simulink model "ofdm_txrx_supermimo_coded"  (Simulink 7.1)
settings: Solver=VariableStepDiscrete  SolverMode=SingleTasking  StartTime=0.0  StopTime=2*simOnly_simLength  RelTol=1e-3
PostLoadFcn:
  | ofdm_rx_supermimo_init
InitFcn:
  | ofdm_rx_supermimo_init
StartFcn:
  | ofdm_rx_supermimo_init
values from neighbouring files (symbols this model uses):
  Action0_Config = 0* 31*2^18 + 0*2^24 + PHY_AUTORESPONSE_REQ_GOODHDR + PHY_AUTORESPONSE_REQ_GOODPKT + PHY_AUTORESPONSE_REQ_FLAGA    [ofdm_tx_supermimo_init.m]
  Action1_Config = 0* 62*2^18 + PHY_AUTORESPONSE_REQ_GOODHDR + PHY_AUTORESPONSE_REQ_GOODPKT    [ofdm_tx_supermimo_init.m]
  Action2_Config = 0    [ofdm_tx_supermimo_init.m]
  Action3_Config = 0    [ofdm_tx_supermimo_init.m]
  Action4_Config = 0    [ofdm_tx_supermimo_init.m]
  Action5_Config = 0    [ofdm_tx_supermimo_init.m]
  ActionID_AFTransmit = 31    [ofdm_tx_supermimo_init.m]
  ActionID_Disabled = 0    [ofdm_tx_supermimo_init.m]
  ActionID_SetFlagA = 62    [ofdm_tx_supermimo_init.m]
  ActionID_SetFlagB = 61    [ofdm_tx_supermimo_init.m]
  BER_RAM_init_size = 2048    [ofdm_tx_supermimo_init.m]
  channel_h = [1 0; 1 0] * 0.3    [ofdm_tx_supermimo_init.m]
  CPLength = 16    [ofdm_tx_supermimo_init.m]
  FEC_Config = fec_coding_en*1 + fec_soft_dec*2 + fec_qpsk_scl*2^4 + fec_16qam_scl*2^8    (= 4195)    [ofdm_tx_supermimo_init.m]
  longCorr_coef_i = [-4*real(LTS) 0]    [ofdm_rx_supermimo_init.m]
  longCorr_coef_q = [4*imag(LTS) 0]    [ofdm_rx_supermimo_init.m]
  Match0_Config = 0    [ofdm_tx_supermimo_init.m]
  Match1_Config = 0    [ofdm_tx_supermimo_init.m]
  Match2_Config = 0    [ofdm_tx_supermimo_init.m]
  Match3_Config = 0    [ofdm_tx_supermimo_init.m]
  Match4_Config = 0    [ofdm_tx_supermimo_init.m]
  Match5_Config = 0    [ofdm_tx_supermimo_init.m]
  Match6_Config = 0    [ofdm_tx_supermimo_init.m]
  Match7_Config = 0    [ofdm_tx_supermimo_init.m]
  max_CP_length = 16    [ofdm_tx_supermimo_init.m]
  max_num_baseRateSymbols = 31    [ofdm_tx_supermimo_init.m]
  max_num_subcarriers = 64    [ofdm_tx_supermimo_init.m]
  max_num_trainingSymbols = 15    [ofdm_tx_supermimo_init.m]
  max_numBytes = 16384    [ofdm_tx_supermimo_init.m]
  max_OFDM_symbols = 1023    [ofdm_tx_supermimo_init.m]
  modConstellation_qam16 = .75*[1 3 -1 -3]./3    [ofdm_tx_supermimo_init.m]
  modConstellation_qam64 = 0.875*[3 1 5 7 -3 -1 -5 -7]./7    [ofdm_tx_supermimo_init.m]
  modConstellation_qpsk = [1 -1]./sqrt(2)    [ofdm_tx_supermimo_init.m]
  numBaseRateSymbols = numBaseRateSymbols *2    [ofdm_tx_supermimo_init.m]
  numSubcarriers = 64    [ofdm_tx_supermimo_init.m]
  numTrainingSymbols = 2    [ofdm_tx_supermimo_init.m]
  pilot_indicies = 7 + ( (64-21) * 2^8) + ( (64-7) * 2^16) + (21 * 2^24)    (= 356068103)    [ofdm_tx_supermimo_init.m]
  pilotValues = hex2dec('A57D') + (2^16 * hex2dec('5A82'))    [ofdm_tx_supermimo_init.m]
  pktByteNums = numHeaderBytes +  				byteIndex_numPayloadBytes(1)*2^8 +  				byteIndex_numPayloadBytes(2)*2^16 +  				byteIndex_simpleDynModMasks * 2^24    [ofdm_rx_supermimo_init.m]
  PktDet_Delay = 58+32 +      Symbol_Timing_Offset*2^7 +      0*2^16 +      (117* 2^24)    (= 1962935642)    [ofdm_rx_supermimo_init.m]
  pktTiming_count_payloadStart = pktTiming_count_lsStart + pktTiming_ls_num*pktTiming_ls_length    [ofdm_rx_supermimo_init.m]
  preamble = preamble_scale*[shortsyms_10 longsyms_2]    [ofdm_tx_supermimo_init.m]
  preamble_ROM = preamble_I_ROM + preamble_Q_ROM*2^16    [ofdm_tx_supermimo_init.m]
  RAM_init_size = max_numBytes    (= 16384)    [ofdm_tx_supermimo_init.m]
  reg_AF_Tx_Blanking = (320+80) + ( (320+80+79)*2^16)    (= 31392144)    [ofdm_rx_supermimo_init.m]
  reg_AF_Tx_Scaling = 2944    [ofdm_rx_supermimo_init.m]
  reg_Interrupt_PktBuf_Control = reg_InterruptControl +  	1 * 2^16 +  	0 * 2^24    (= 65536)    [ofdm_rx_supermimo_init.m]
  reg_PilotCalcParams = (4 * 2^16) + (minPilotChanMag)    (= 262184.96)    [ofdm_rx_supermimo_init.m]
  regRx_chanEst_minMag = ((0.5)*2^15) + ((0.5 * 2^15)*2^16)    (= 1073758208)    [ofdm_rx_supermimo_init.m]
  regRx_coarseCalcCorrection = round((1.3e-3 - 1.2982e-3)*2^32)    [ofdm_rx_supermimo_init.m]
  regRx_pilotCalcCorrection = round(1.00115 * 2^31)    [ofdm_rx_supermimo_init.m]
  regRx_preCFOoptions = 0 * 2^0 +  	1 * 2^1 +  	0    (= 2)    [ofdm_rx_supermimo_init.m]
  rx_controlBits = 1 * 2  		 + 1 * 4  		 + 0 * 8  		 + 1 * 16 * rx_SISO_Mode  		 + 1 * 32  		 + 1 * 64  		 + 1 * 128  		 + 1 * 256  		 + 1 * 512  		 + 1 * 1024  		 + 1 * 4096  		 + 0 * 16384  		 + 0 * 32768  		 + 1 * 65536  		 + 0 * 131072  		 + 0 * 262144  		 + 1 * 524288  		 + 1 * 1048576 * tx_Alamouti_Mode  		 + 0 * 2097152           + 1 * 33554432  		 + 0 * 4194304  		 + 0 * 8388608  		 + 0 * 16777216  		 + 1 *  ...(+49 chars)    [ofdm_rx_supermimo_init.m]
  Rx_PktDet_LongCorr_Params = LongCorrCounterLoad + 0*2^8 + 0*2^16 + 255*2^24    (= 4278190325)    [ofdm_rx_supermimo_init.m]
  Rx_PktDet_LongCorrThresholds = 8000    [ofdm_rx_supermimo_init.m]
  rx_postEq_scaling = round(rxScaling*2^11) * (1 + 2^16)    [ofdm_rx_supermimo_init.m]
  RxDataScrambling_Seq = [40 198 78 63 82 173 102 245 48 111 172 115 147 230 216 93 72 65 62 2 205 242 122 90 128 83 105 97 73 10 5 252]    [ofdm_tx_supermimo_init.m]
  RxReg_FixedPktLen = 0    [ofdm_rx_supermimo_init.m]
  rxReg_pktDetCorr = 2^00 * (floor(0.7*128)) +  	2^08 * (300) +  	0    [ofdm_rx_supermimo_init.m]
  shortSymbol_time = shortSymbol_time(1:16).'    [ofdm_tx_supermimo_init.m]
  simOnly_numSamples = length(preamble)+( (numSubcarriers+CPLength)*(numTrainingSymbols + numBaseRateSymbols + numFullRateSymbols/2) )    [ofdm_tx_supermimo_init.m]
  train = [train train]    [ofdm_tx_supermimo_init.m]
  tx_controlBits = (antB_preambleShift * 2^4) + (2^1 * tx_Alamouti_Mode) +  	(2^0 * tx_SISO_Mode) + 4*0 + 8*1 + 0*256 + 512*0 + 1024*0 +  	0*hex2dec('1000') + 0*hex2dec('2000') + 0*hex2dec('4000') +  	1*hex2dec('8000') + 1*hex2dec('10000') + hex2dec('20000') +  	0*hex2dec('100000')    [ofdm_tx_supermimo_init.m]
  tx_delays = (50*2^0) + (0*2^8) + (30*2^12)    (= 122930)    [ofdm_tx_supermimo_init.m]
  tx_scaling = (3072) + (12288 * 2^16)    (= 805309440)    [ofdm_tx_supermimo_init.m]
  TxDataScrambling_Seq = [40 198 78 63 82 173 102 245 48 111 172 115 147 230 216 93 72 65 62 2 205 242 122 90 128 83 105 97 73 10 5 252]    [ofdm_tx_supermimo_init.m]
  txReg_symbolCounts = (2^8 *numBaseRateSymbols ) + numTrainingSymbols    [ofdm_tx_supermimo_init.m]
  TxRx_FFTScaling = (3 * 2^0) +  	(2 * 2^2) +  	(1 * 2^4) +  	(1 * 2^6) +  	(1 * 2^8) +  	(0 * 2^10)    (= 347)    [ofdm_tx_supermimo_init.m]
undefined symbols (not defined in the file or in its folder): AntA, AntB, BaseRate, Down, gcbh, numBuffs, numBytes, Up, xlBlockLoadCallback, xlGetSimulinkPeriod, xlipgetpartsetting
libraries: xbsIndex_r4 x6000, simulink x4, dspsigops x4, commchan3 x2, dspsrcs4 x2, commrflib2 x2
(part 19 of 63)

== system ofdm_txrx_supermimo_coded/OFDM Tx MIMO/Pre-Spin,  Filters & DACs/Interpolation ==
  note: Filters are implemented in external
      | rate_change_filters pcore
  "A_I " : Inport
  "A_Q " : Inport  Port=2
  "B_I " : Inport  Port=3
  "B_Q " : Inport  Port=4
  Tx_AntA_I_DIV1 : xbsIndex_r4/Gateway In  arith_type="Signed  (2's comp)"  n_bits=16  bin_pt=15  quantization="Round  (unbiased: +/- Inf)"  overflow=Saturate  period=1  dbl_ovrd=off  timing_constraint=None  locs_specified=off  LOCs={}  block_type=gatewayin
      settings: infoedit="Gateway in block.  Converts inputs of type Simulink integer, double and fixed point to  Xilinx fixed point type.<P><P>Hardware notes:  In hardware these blocks become top level input ports."  block_version=10.1.3
  Tx_AntA_I_DIV4 : xbsIndex_r4/Gateway Out  hdl_port=on  timing_constraint=None  locs_specified=off  LOCs={}  block_type=gatewayout
      settings: infoedit="Gateway out block.  Converts Xilinx fixed point inputs into ouputs of type Simulink integer, double, or fixed point.<P><P>Hardware notes:  In hardware these blocks become top level output ports or are discarded, depending on how they are configured."  block_version=10.1.3
  Tx_AntA_Q_DIV1 : xbsIndex_r4/Gateway In  arith_type="Signed  (2's comp)"  n_bits=16  bin_pt=15  quantization="Round  (unbiased: +/- Inf)"  overflow=Saturate  period=1  dbl_ovrd=off  timing_constraint=None  locs_specified=off  LOCs={}  block_type=gatewayin
      settings: infoedit="Gateway in block.  Converts inputs of type Simulink integer, double and fixed point to  Xilinx fixed point type.<P><P>Hardware notes:  In hardware these blocks become top level input ports."  block_version=10.1.3
  Tx_AntA_Q_DIV4 : xbsIndex_r4/Gateway Out  hdl_port=on  timing_constraint=None  locs_specified=off  LOCs={}  block_type=gatewayout
      settings: infoedit="Gateway out block.  Converts Xilinx fixed point inputs into ouputs of type Simulink integer, double, or fixed point.<P><P>Hardware notes:  In hardware these blocks become top level output ports or are discarded, depending on how they are configured."  block_version=10.1.3
  Tx_AntB_I_DIV1 : xbsIndex_r4/Gateway In  arith_type="Signed  (2's comp)"  n_bits=16  bin_pt=15  quantization="Round  (unbiased: +/- Inf)"  overflow=Saturate  period=1  dbl_ovrd=off  timing_constraint=None  locs_specified=off  LOCs={}  block_type=gatewayin
      settings: infoedit="Gateway in block.  Converts inputs of type Simulink integer, double and fixed point to  Xilinx fixed point type.<P><P>Hardware notes:  In hardware these blocks become top level input ports."  block_version=10.1.3
  Tx_AntB_I_DIV4 : xbsIndex_r4/Gateway Out  hdl_port=on  timing_constraint=None  locs_specified=off  LOCs={}  block_type=gatewayout
      settings: infoedit="Gateway out block.  Converts Xilinx fixed point inputs into ouputs of type Simulink integer, double, or fixed point.<P><P>Hardware notes:  In hardware these blocks become top level output ports or are discarded, depending on how they are configured."  block_version=10.1.3
  Tx_AntB_Q_DIV1 : xbsIndex_r4/Gateway In  arith_type="Signed  (2's comp)"  n_bits=16  bin_pt=15  quantization="Round  (unbiased: +/- Inf)"  overflow=Saturate  period=1  dbl_ovrd=off  timing_constraint=None  locs_specified=off  LOCs={}  block_type=gatewayin
      settings: infoedit="Gateway in block.  Converts inputs of type Simulink integer, double and fixed point to  Xilinx fixed point type.<P><P>Hardware notes:  In hardware these blocks become top level input ports."  block_version=10.1.3
  Tx_AntB_Q_DIV4 : xbsIndex_r4/Gateway Out  hdl_port=on  timing_constraint=None  locs_specified=off  LOCs={}  block_type=gatewayout
      settings: infoedit="Gateway out block.  Converts Xilinx fixed point inputs into ouputs of type Simulink integer, double, or fixed point.<P><P>Hardware notes:  In hardware these blocks become top level output ports or are discarded, depending on how they are configured."  block_version=10.1.3
  A_I : Outport
  A_Q : Outport  Port=2
  B_I : Outport  Port=3
  B_Q : Outport  Port=4
  wires:
  Tx_AntA_I_DIV4/1 -> Tx_AntA_I_DIV1/1
  Tx_AntA_Q_DIV4/1 -> Tx_AntA_Q_DIV1/1
  Tx_AntB_I_DIV4/1 -> Tx_AntB_I_DIV1/1
  Tx_AntB_Q_DIV4/1 -> Tx_AntB_Q_DIV1/1
  "A_I "/1 -> Tx_AntA_I_DIV4/1
  "A_Q "/1 -> Tx_AntA_Q_DIV4/1
  Tx_AntA_I_DIV1/1 -> A_I/1
  Tx_AntA_Q_DIV1/1 -> A_Q/1
  Tx_AntB_I_DIV1/1 -> B_I/1
  "B_I "/1 -> Tx_AntB_I_DIV4/1
  Tx_AntB_Q_DIV1/1 -> B_Q/1
  "B_Q "/1 -> Tx_AntB_Q_DIV4/1

== system ofdm_txrx_supermimo_coded/OFDM Tx MIMO/Pre-Spin,  Filters & DACs/Pre-CFO Sinusoid ==
  Constant1 : xbsIndex_r4/Constant  arith_type=Signed (2's comp)  const=0  n_bits=16  bin_pt=15  explicit_period=on  period=4  equ=P=C  opselect=C  inp2=PCIN>>17  opr=+  inp1=P  carry=CIN  dbl_ovrd=off  block_type=constant
      settings: block_version=VER_STRING_GOES_HERE
  Constant2 : xbsIndex_r4/Constant  arith_type=Signed (2's comp)  const=1  n_bits=16  bin_pt=15  explicit_period=on  period=4  equ=P=C  opselect=C  inp2=PCIN>>17  opr=+  inp1=P  carry=CIN  dbl_ovrd=off  block_type=constant
      settings: block_version=VER_STRING_GOES_HERE
  Convert1 : xbsIndex_r4/Convert  arith_type=Unsigned  n_bits=1  bin_pt=0  quantization=Truncate  overflow=Wrap  latency=0  dbl_ovrd=off  pipeline=off  block_type=convert
      settings: xl_area=[0, 0, 0, 0, 0, 0, 0]  block_version=VER_STRING_GOES_HERE
  Down Sample : xbsIndex_r4/Down Sample  sample_ratio=2  sample_phase="Last Value of Frame  (most efficient)"  en=off  latency=1  dbl_ovrd=off  block_type=dsamp
      settings: block_version=VER_STRING_GOES_HERE
  Logical1 : xbsIndex_r4/Logical  logical_function=OR  inputs=2  en=off  latency=0  precision=Full  arith_type=Unsigned  n_bits=8  bin_pt=2  align_bp=on  dbl_ovrd=off  block_type=logical
      settings: xl_area=[1 0 0 1 0 0 0]  block_version=VER_STRING_GOES_HERE
  Mux1 : xbsIndex_r4/Mux  inputs=2  en=off  latency=0  precision=Full  arith_type=Unsigned  n_bits=16  bin_pt=14  quantization=Truncate  overflow=Wrap  dbl_ovrd=off  block_type=mux
      settings: block_version=VER_STRING_GOES_HERE
  Mux3 : xbsIndex_r4/Mux  inputs=2  en=off  latency=0  precision=Full  arith_type=Unsigned  n_bits=16  bin_pt=14  quantization=Truncate  overflow=Wrap  dbl_ovrd=off  block_type=mux
      settings: block_version=VER_STRING_GOES_HERE
  I : Outport
  Q : Outport  Port=2
  wires:
  [RxAutoReplyProc_UsePreCFO] -> Convert1/1
  [CFO_DDSOut_I] -> Mux1/3
  [CFO_DDSOut_Q] -> Mux3/3
  Down Sample/1 -> Mux3/1, Mux1/1
  Constant2/1 -> Mux1/2
  Constant1/1 -> Mux3/2
  Logical1/1 -> Down Sample/1
  [regTx_AlwaysUsePreSpin] -> Logical1/1
  Convert1/1 -> Logical1/2
  Mux1/1 -> I/1
  Mux3/1 -> Q/1

== system ofdm_txrx_supermimo_coded/OFDM Tx MIMO/Pre-Spin,  Filters & DACs/Scaling ==
  note: Delay for mults (2)
      | and filters (6)
  Payload : Inport  OutDataType=sfix(16)  OutScaling=2^0
  "A_I " : Inport  Port=2
  "A_Q " : Inport  Port=3
  "B_I " : Inport  Port=4
  "B_Q " : Inport  Port=5
  Delay1 : xbsIndex_r4/Delay  en=off  latency=8  dbl_ovrd=off  reg_retiming=off  block_type=delay
  Mult{1..3,7} (4 alike) : xbsIndex_r4/Mult  precision=User Defined  arith_type="Signed  (2's comp)"  n_bits=16  bin_pt=15  quantization=Truncate  overflow=Saturate  en=off  latency=1  dbl_ovrd=off  use_behavioral_HDL=off  use_embedded=on  opt=Speed  optimum_pipeline=off  pipeline=on  use_rpm=on  placement_style=Triangular  block_type=mult
      settings: infoedit=Hardware notes: To use the internal pipeline stage of the dedicated multiplier you must select 'Pipeline for maximum performance'.  block_version=10.1.3
  Mux4 : xbsIndex_r4/Mux  inputs=2  en=off  latency=0  precision=Full  arith_type=Unsigned  n_bits=16  bin_pt=14  quantization=Truncate  overflow=Wrap  dbl_ovrd=off  block_type=mux
      settings: block_version=VER_STRING_GOES_HERE
  Up Sample13 : xbsIndex_r4/Up Sample  sample_ratio=2  copy_samples=on  dbl_ovrd=off  block_type=usamp
      settings: infoedit="Up samples input data.  Inserted values  can be zeros or copies of the most recent input sample.<P><P>Hardware notes: No hardware is needed if inserted values are copies of the input sample; otherwise, a mux and single bit flip-flop are used."  block_version=10.1.3
  A_I : Outport
  A_Q : Outport  Port=2
  B_I : Outport  Port=3
  B_Q : Outport  Port=4
  wires:
  Delay1/1 -> Up Sample13/1
  Up Sample13/1 -> Mux4/1
  Mux4/1 -> Mult2/1, Mult3/1, Mult1/1, Mult7/1
  [TxReg_postIFFTScaling] -> Mux4/3
  [TxReg_PreambleScaling] -> Mux4/2
  Payload/1 -> Delay1/1
  "A_I "/1 -> Mult7/2
  "A_Q "/1 -> Mult1/2
  Mult7/1 -> A_I/1
  Mult1/1 -> A_Q/1
  Mult2/1 -> B_I/1
  "B_I "/1 -> Mult2/2
  Mult3/1 -> B_Q/1
  "B_Q "/1 -> Mult3/2

== system ofdm_txrx_supermimo_coded/OFDM Tx MIMO/Training_Data/Ant Selector ==
  note: In SISO and multiplexing modes, antenna selction should switch with
      | alternate OFDM symbols during full-rate paylod symbols. During training
      | and base rate symbols there is no need to switch.
      | In Alamouti mode, the selection switches during base-rate
      | and full-rate symbols.
  BaseRate : Inport  OutDataType=sfix(16)  OutScaling=2^0
  CP#_Data : Inport  Port=2  OutDataType=sfix(16)  OutScaling=2^0
  Rst : Inport  Port=3  OutDataType=sfix(16)  OutScaling=2^0
  Inverter2 : xbsIndex_r4/Inverter  en=off  latency=0  dbl_ovrd=off  block_type=inv
      settings: block_version=VER_STRING_GOES_HERE
  Logical1 : xbsIndex_r4/Logical  logical_function=AND  inputs=2  en=off  latency=0  precision=Full  arith_type=Unsigned  n_bits=16  bin_pt=0  align_bp=on  dbl_ovrd=off  block_type=logical
      settings: block_version=VER_STRING_GOES_HERE
  Logical2 : xbsIndex_r4/Logical  logical_function=OR  inputs=2  en=off  latency=0  precision=Full  arith_type=Unsigned  n_bits=16  bin_pt=0  align_bp=on  dbl_ovrd=off  block_type=logical
      settings: block_version=VER_STRING_GOES_HERE
  Oscillator : Subsystem
  negedge : Subsystem
  Sel : Outport  OutDataType=sfix(16)  OutScaling=2^0
  wires:
  CP#_Data/1 -> negedge/1(D)
  Oscillator/1(Q) -> Sel/1
  negedge/1(Q) -> Logical1/2
  Inverter2/1 -> Logical2/2
  Rst/1 -> Oscillator/1(rst)
  BaseRate/1 -> Inverter2/1
  Logical1/1 -> Oscillator/2(en)
  [TxReg_Alamouti_Mode] -> Logical2/1
  Logical2/1 -> Logical1/1

== system ofdm_txrx_supermimo_coded/OFDM Tx MIMO/Training_Data/FlexibleMod ==
  note: Latency = 2?
  note: Latency = 1
  note: Latency = 0
  ifft_index : Inport  OutDataType=sfix(16)  OutScaling=2^0
  packet_done : Inport  Port=2  OutDataType=sfix(16)  OutScaling=2^0
  vin : Inport  Port=3  OutDataType=sfix(16)  OutScaling=2^0
  start : Inport  Port=4  OutDataType=sfix(16)  OutScaling=2^0
  BaseRate_En : Inport  Port=5  OutDataType=sfix(16)  OutScaling=2^0
  ant_sel : Inport  Port=6  OutDataType=sfix(16)  OutScaling=2^0
  AddSub : xbsIndex_r4/AddSub  mode=Addition  use_carryin=off  use_carryout=off  en=off  latency=0  precision=User Defined  arith_type=Unsigned  n_bits=16  bin_pt=0  quantization=Truncate  overflow=Wrap  dbl_ovrd=off  use_behavioral_HDL=on  pipelined=off  use_rpm=on  hw_selection=Fabric  block_type=addsub
      settings: xl_area=[2 0 0 4 0 0 0]  block_version=VER_STRING_GOES_HERE
  Assert4 : xbsIndex_r4/Assert  assert_type=on  type_source=Explicitly  arith_type=Unsigned  n_bits=16  bin_pt=0  assert_rate=off  rate_source=Explicitly  period=2  output_port=on  block_type=assert
      settings: infoedit=Asserts a user-defined sample rate and/or type on a signal.<P><P>Hardware notes: In hardware this block costs nothing.  block_version=VER_STRING_GOES_HERE
  Constant3 : xbsIndex_r4/Constant  arith_type=Unsigned  const=2  n_bits=2  bin_pt=0  explicit_period=off  period=1  equ=P=C  opselect=C  inp2=PCIN>>17  opr=+  inp1=P  carry=CIN  dbl_ovrd=off  block_type=constant
      settings: block_version=VER_STRING_GOES_HERE
  Display : Display
  Display1 : Display
  Encoder : Scope  NumInputPorts=11
      settings: TimeRange=13320  YMin=0~-1~-1~0~-1~0~0~0~-1~100~-1  YMax=1~2~2~250~2~150~300~200~2~300~2  SaveName=ScopeData31
  FEC1 : Scope  NumInputPorts=7
      settings: TimeRange=15000  YMin=0~0~-1~-5~-1~-5~-1  YMax=2~2~2~5~2~5~2  SaveName=ScopeData32
  Gateway Out{1..18} (18 alike) : xbsIndex_r4/Gateway Out  hdl_port=off  timing_constraint=None  locs_specified=off  LOCs={}  block_type=gatewayout
      settings: infoedit="Gateway out block.  Converts Xilinx fixed point inputs into ouputs of type Simulink integer, double, or fixed point.<P><P>Hardware notes:  In hardware these blocks become top level output ports or are discarded, depending on how they are configured."  block_version=10.1.3
  Inverter : xbsIndex_r4/Inverter  en=off  latency=0  dbl_ovrd=off  block_type=inv
  Logical2 : xbsIndex_r4/Logical  logical_function=OR  inputs=2  en=off  latency=0  precision=Full  arith_type=Unsigned  n_bits=8  bin_pt=2  align_bp=on  dbl_ovrd=off  block_type=logical
      settings: xl_area=[1 0 0 1 0 0 0]  block_version=VER_STRING_GOES_HERE
  PktBuffer_CRC1 : Subsystem  Orientation=left
  QAM : Subsystem
  Register1 : xbsIndex_r4/Register  init=0  rst=off  en=off  dbl_ovrd=off  block_type=register
      settings: xl_area=[2 4 0 0 0 0 0]  block_version=VER_STRING_GOES_HERE
  Register2 : xbsIndex_r4/Register  init=0  rst=off  en=off  dbl_ovrd=off  block_type=register
      settings: xl_area=[2 4 0 0 0 0 0]  block_version=VER_STRING_GOES_HERE
  Relational4 : xbsIndex_r4/Relational  mode=a<=b  en=off  latency=0  dbl_ovrd=off  block_type=relational
      settings: xl_area=[0, 0, 0, 0, 0, 0, 0]  block_version=VER_STRING_GOES_HERE
  S-R Latch1 : Subsystem
  SR Latch : Subsystem
  control : Subsystem
  data_buffer : Subsystem
  fec_encoder : xbsIndex_r4/Black Box  init_code=fec_encoder_config  sim_method=ISE Simulator  block_type=blackbox2
      settings: infoedit="Incorporates black box HDL and simulation model into a System Generator design.<P><P>You must supply a Black Box with certain information about the HDL component you would like to bring into System Generator. This information is provided through a Matlab function.<P><P>When \"Simulation mode\" is set to \"Inactive\", you will typically want to provide a separate simulation model by using a Simulation Multiplexer.<P>When \"Simulation mode\" is set to \"External co-simulator\", you must include a ModelSim block in the design."  block_version=10.1.3
  posedge1 : Subsystem  mask{period=1}
  posedge2 : Subsystem  Orientation=left  mask{period=1}
  slicer : Subsystem
  I : Outport  OutDataType=sfix(16)  OutScaling=2^0
  Q : Outport  Port=2  OutDataType=sfix(16)  OutScaling=2^0
  Payload Done : Outport  Port=3  OutDataType=sfix(16)  OutScaling=2^0
  wires:
  control/3(mod) -> data_buffer/4(mod), Register1/1
  vin/1 -> control/6(valid)
  control/2(index) -> data_buffer/3(index), Register2/1
  slicer/1(Byte Out) -> QAM/1(Mod Bits)
  QAM/1(I) -> I/1
  QAM/2(Q) -> Q/1
  data_buffer/3(16-Bit Register) -> slicer/1(16-Bit In)
  control/1(reset) -> data_buffer/2(reset)
  ifft_index/1 -> control/4(ifft_index), Gateway Out8/1
  BaseRate_En/1 -> control/5(BaseRate_En)
  ant_sel/1 -> control/7(ant_sel), Gateway Out11/1
  posedge1/1(pulse) -> control/3(ready), PktBuffer_CRC1/1(Rst), SR Latch/1(R)
  PktBuffer_CRC1/2(FullRateMasks) -> control/1(FullRateMasks)
  Register2/1 -> slicer/2(index)
  Register1/1 -> QAM/2(Mod Sel)
  Relational4/1 -> SR Latch/2(S)
  SR Latch/1(Q) -> Gateway Out14/1, Payload Done/1, Gateway Out18/1, Gateway Out13/1, posedge2/1(signal)
  S-R Latch1/1(Q) -> posedge1/1(signal)
  Logical2/1 -> S-R Latch1/1(R)
  start/1 -> S-R Latch1/2(S), Gateway Out1/1, fec_encoder/2, Gateway Out10/1
  [TxReset] -> Logical2/2, Inverter/1
  packet_done/1 -> Logical2/1, Gateway Out12/1
  Constant3/1 -> AddSub/1
  AddSub/1 -> Relational4/1
  data_buffer/2(RAM addr Vout) -> Gateway Out9/1, Gateway Out2/1, fec_encoder/5
  fec_encoder/3 -> PktBuffer_CRC1/3(DataOut_Addr), Gateway Out6/1
  PktBuffer_CRC1/3(Dout) -> fec_encoder/7, Gateway Out7/1
  fec_encoder/4 -> data_buffer/1(RAM data), Gateway Out3/1
  fec_encoder/2 -> PktBuffer_CRC1/2(DataOut_Addr Vin), Gateway Out5/1
  data_buffer/1(RAM address (B)) -> Relational4/2
  Inverter/1 -> Gateway Out4/1, fec_encoder/1
  Gateway Out4/1 -> Encoder/1  "nrst"
  Gateway Out1/1 -> Encoder/2  "start"
  Gateway Out2/1 -> Encoder/3  "fec_rd"
  Gateway Out3/1 -> Encoder/4  "fec_data"
  Gateway Out5/1 -> Encoder/5  "info_rd"
  Gateway Out6/1 -> Encoder/6  "info_raddr"
  Gateway Out7/1 -> Encoder/7  "info_scram"
  Gateway Out8/1 -> FEC1/1  "ifft_index"
  Gateway Out9/1 -> FEC1/2  "vout"
  Gateway Out10/1 -> FEC1/3  "start"
  Gateway Out11/1 -> FEC1/4  "ant_sel"
  Gateway Out12/1 -> FEC1/5  "pkt_done"
  Gateway Out13/1 -> FEC1/6  "nbytes"
  Gateway Out14/1 -> FEC1/7  "payload_done"
  PktBuffer_CRC1/1(NumBytes) -> Assert4/1
  fec_encoder/1 -> AddSub/2, control/2(length), Display/1, Gateway Out17/1
  PktBuffer_CRC1/4(Info_data) -> fec_encoder/6, Gateway Out15/1
  [Coding_En] -> fec_encoder/3, Gateway Out16/1
  Gateway Out15/1 -> Encoder/8  "info_data"
  Gateway Out16/1 -> Encoder/9  "coding_en"
  Gateway Out17/1 -> Encoder/10  "codeword_len"
  Gateway Out18/1 -> Encoder/11  "pkt_done"
  Assert4/1 -> fec_encoder/8, Display1/1
  posedge2/1(pulse) -> fec_encoder/4

== system ofdm_txrx_supermimo_coded/OFDM Tx MIMO/Training_Data/Training_Pilots ==
  note: The training seq lookup tables have latency=3 to
      | align their output with the IFFT's expected inputs.
      | The IFFT xn output runs three cycles ahead of the input
      | data (i.e. xn=3 when data[0] should be on the IFFT inputs.
  Xn_index : Inport  OutDataType=sfix(16)  OutScaling=2^0
  I_mod : Inport  Port=2  OutDataType=sfix(16)  OutScaling=2^0
  Q_mod : Inport  Port=3  OutDataType=sfix(16)  OutScaling=2^0
  train# : Inport  Port=4  OutDataType=sfix(16)  OutScaling=2^0
  antSel : Inport  Port=5  OutDataType=sfix(16)  OutScaling=2^0
  rst : Inport  Port=6  OutDataType=sfix(16)  OutScaling=2^0
  Concat : xbsIndex_r4/Concat  num_inputs=2  dbl_ovrd=off  block_type=concat
      settings: block_version=VER_STRING_GOES_HERE
  Convert : xbsIndex_r4/Convert  arith_type=Unsigned  n_bits=1  bin_pt=0  quantization=Truncate  overflow=Wrap  latency=0  dbl_ovrd=off  pipeline=off  block_type=convert
      settings: xl_area=[0, 0, 0, 0, 0, 0, 0]  block_version=VER_STRING_GOES_HERE
  Delay1 : xbsIndex_r4/Delay  en=off  latency=3  dbl_ovrd=off  reg_retiming=off  block_type=delay
      settings: infoedit=Hardware notes: A delay line is a chain, each link of which is an SRL16 followed by a flip-flop. If register retiming is enabled, the delay line is a chain of flip-flops.  xl_area=[1 1 0 0 0 0 0]  block_version=VER_STRING_GOES_HERE
  Inverter : xbsIndex_r4/Inverter  en=off  latency=0  dbl_ovrd=off  block_type=inv
      settings: xl_area=[0, 0, 0, 0, 0, 0, 0]  block_version=VER_STRING_GOES_HERE
  Mux : xbsIndex_r4/Mux  inputs=2  en=off  latency=0  precision=User Defined  arith_type="Signed  (2's comp)"  n_bits=16  bin_pt=15  quantization=Truncate  overflow=Wrap  dbl_ovrd=off  block_type=mux
      settings: xl_area=[0, 0, 0, 0, 0, 0, 0]  block_version=VER_STRING_GOES_HERE
  Mux1 : xbsIndex_r4/Mux  inputs=2  en=off  latency=0  precision=User Defined  arith_type="Signed  (2's comp)"  n_bits=16  bin_pt=15  quantization=Truncate  overflow=Wrap  dbl_ovrd=off  block_type=mux
      settings: xl_area=[0, 0, 0, 0, 0, 0, 0]  block_version=VER_STRING_GOES_HERE
  Scrambled_Pilot_Inserter1 : Subsystem
  Train_I : xbsIndex_r4/ROM  depth=2*numSubcarriers  initVector=real(train)  distributed_mem=Block RAM  rst=off  init_reg=0  en=on  latency=3  arith_type="Signed  (2's comp)"  n_bits=modConstellation_prec  bin_pt=modConstellation_bp  dbl_ovrd=off  optimize=Area  use_rpm=off  block_type=sprom
      settings: xl_area=[0, 0, 0, 0, 0, 0, 0]  block_version=VER_STRING_GOES_HERE
  Train_Q : xbsIndex_r4/ROM  depth=2*numSubcarriers  initVector=imag(train)  distributed_mem=Block RAM  rst=off  init_reg=0  en=on  latency=3  arith_type="Signed  (2's comp)"  n_bits=modConstellation_prec  bin_pt=modConstellation_bp  dbl_ovrd=off  optimize=Area  use_rpm=off  block_type=sprom
      settings: xl_area=[0, 0, 0, 0, 0, 0, 0]  block_version=VER_STRING_GOES_HERE
  tx_I : Outport  OutDataType=sfix(16)  OutScaling=2^0
  tx_Q : Outport  Port=2  OutDataType=sfix(16)  OutScaling=2^0
  wires:
  Convert/1 -> Delay1/1
  Mux1/1 -> tx_Q/1
  Mux/1 -> tx_I/1
  Scrambled_Pilot_Inserter1/1(" I") -> Mux/3
  Scrambled_Pilot_Inserter1/2(" Q") -> Mux1/3
  Train_I/1 -> Mux/2
  Train_Q/1 -> Mux1/2
  Inverter/1 -> Train_I/2, Train_Q/2
  Concat/1 -> Train_Q/1, Train_I/1
  Xn_index/1 -> Concat/2, Scrambled_Pilot_Inserter1/1(IFFT Index)
  train#/1 -> Inverter/1, Convert/1, Scrambled_Pilot_Inserter1/6(Train#)
  antSel/1 -> Concat/1, Scrambled_Pilot_Inserter1/5(Ant Sel)
  I_mod/1 -> Scrambled_Pilot_Inserter1/2("I ")
  Q_mod/1 -> Scrambled_Pilot_Inserter1/3("Q ")
  Delay1/1 -> Mux/1, Mux1/1
  rst/1 -> Scrambled_Pilot_Inserter1/4(rst)

== system ofdm_txrx_supermimo_coded/OFDM Tx MIMO/TxControl/2x ==
  I : Inport  OutDataType=sfix(16)  OutScaling=2^0
  Concat1 : xbsIndex_r4/Concat  num_inputs=2  dbl_ovrd=off  block_type=concat
      settings: block_version=VER_STRING_GOES_HERE
  Constant : xbsIndex_r4/Constant  arith_type=Unsigned  const=0  n_bits=1  bin_pt=0  explicit_period=off  period=1  equ=P=C  opselect=C  inp2=PCIN>>17  opr=+  inp1=P  carry=CIN  dbl_ovrd=off  block_type=constant
      settings: block_version=VER_STRING_GOES_HERE
  O : Outport  OutDataType=sfix(16)  OutScaling=2^0
  wires:
  Constant/1 -> Concat1/2
  I/1 -> Concat1/1
  Concat1/1 -> O/1

== system ofdm_txrx_supermimo_coded/OFDM Tx MIMO/TxControl/2x1 ==
  I : Inport  OutDataType=sfix(16)  OutScaling=2^0
  Concat1 : xbsIndex_r4/Concat  num_inputs=2  dbl_ovrd=off  block_type=concat
      settings: block_version=VER_STRING_GOES_HERE
  Constant : xbsIndex_r4/Constant  arith_type=Unsigned  const=0  n_bits=1  bin_pt=0  explicit_period=off  period=1  equ=P=C  opselect=C  inp2=PCIN>>17  opr=+  inp1=P  carry=CIN  dbl_ovrd=off  block_type=constant
      settings: block_version=VER_STRING_GOES_HERE
  O : Outport  OutDataType=sfix(16)  OutScaling=2^0
  wires:
  Concat1/1 -> O/1
  I/1 -> Concat1/1
  Constant/1 -> Concat1/2

== system ofdm_txrx_supermimo_coded/OFDM Tx MIMO/TxControl/FFT Start Gen ==
  note: First two symbols are always training and should be processed immediately, back-to-back. The same IFFT
      | output will be repeated numTrainingSyms times. After this, the IFFT processes the base-rate symbols. Because
      | each base-rate symbol is transmitted from both antennas, the IFFT waits a full output symbol period before processing
      | the next symbol. After the base rate symbols, the multiplexing of full-rate symbols begins, so the IFFT is triggered twice
      | per output symbol period (computing two output symbols- one per antenna- per period).
  note: In Alamouti mode, treat base rate symbols
      | same as full rate
  SymNum : Inport  OutDataType=sfix(16)  OutScaling=2^0
  AddSub1 : xbsIndex_r4/AddSub  mode=Addition  use_carryin=off  use_carryout=off  en=off  latency=0  precision=Full  arith_type=Unsigned  n_bits=10  bin_pt=0  quantization=Truncate  overflow=Wrap  dbl_ovrd=off  use_behavioral_HDL=on  pipelined=off  use_rpm=on  hw_selection=Fabric  block_type=addsub
      settings: xl_area=[0, 0, 0, 0, 0, 0, 0]  block_version=VER_STRING_GOES_HERE
  Constant9 : xbsIndex_r4/Constant  arith_type=Unsigned  const=1  n_bits=1  bin_pt=0  explicit_period=off  period=1  equ=P=C  opselect=C  inp2=PCIN>>17  opr=+  inp1=P  carry=CIN  dbl_ovrd=off  block_type=constant
      settings: block_version=VER_STRING_GOES_HERE
  Inverter : xbsIndex_r4/Inverter  en=off  latency=0  dbl_ovrd=off  block_type=inv
      settings: block_version=VER_STRING_GOES_HERE
  LSB : xbsIndex_r4/Slice  nbits=1  boolean_output=on  mode=Lower Bit Location + Width  bit1=0  base1=LSB of Input  bit0=0  base0=LSB of Input  dbl_ovrd=off  block_type=slice
      settings: infoedit="Extracts a given range of bits from each input sample and presents it at the output.  The output type is ordinarily unsigned with binary point at zero, but can be Boolean when the slice is one bit wide.<P><P>Hardware notes: In hardware this block costs nothing."  block_version=VER_STRING_GOES_HERE
  Logical : xbsIndex_r4/Logical  logical_function=OR  inputs=3  en=off  latency=0  precision=Full  arith_type=Unsigned  n_bits=16  bin_pt=0  align_bp=on  dbl_ovrd=off  block_type=logical
      settings: block_version=VER_STRING_GOES_HERE
  Logical1 : xbsIndex_r4/Logical  logical_function=AND  inputs=3  en=off  latency=0  precision=Full  arith_type=Unsigned  n_bits=16  bin_pt=0  align_bp=on  dbl_ovrd=off  block_type=logical
      settings: block_version=VER_STRING_GOES_HERE
  Logical2 : xbsIndex_r4/Logical  logical_function=OR  inputs=2  en=off  latency=0  precision=Full  arith_type=Unsigned  n_bits=16  bin_pt=0  align_bp=on  dbl_ovrd=off  block_type=logical
      settings: block_version=VER_STRING_GOES_HERE
  Logical3 : xbsIndex_r4/Logical  logical_function=AND  inputs=2  en=off  latency=0  precision=Full  arith_type=Unsigned  n_bits=16  bin_pt=0  align_bp=on  dbl_ovrd=off  block_type=logical
      settings: block_version=VER_STRING_GOES_HERE
  Relational1 : xbsIndex_r4/Relational  mode=a>=b  en=off  latency=0  dbl_ovrd=off  block_type=relational
      settings: xl_area=[2 0 0 4 0 0 0]  block_version=VER_STRING_GOES_HERE
  Relational2 : xbsIndex_r4/Relational  mode=a<b  en=off  latency=0  dbl_ovrd=off  block_type=relational
      settings: xl_area=[2 0 0 4 0 0 0]  block_version=VER_STRING_GOES_HERE
  Relational3 : xbsIndex_r4/Relational  mode=a>=b  en=off  latency=0  dbl_ovrd=off  block_type=relational
      settings: xl_area=[2 0 0 4 0 0 0]  block_version=VER_STRING_GOES_HERE
  Relational9 : xbsIndex_r4/Relational  mode=a<=b  en=off  latency=0  dbl_ovrd=off  block_type=relational
      settings: xl_area=[2 0 0 4 0 0 0]  block_version=VER_STRING_GOES_HERE
  En : Outport  OutDataType=sfix(16)  OutScaling=2^0
  wires:
  Constant9/1 -> Relational9/2
  SymNum/1 -> Relational9/1, Relational1/1, Relational2/1, LSB/1, Relational3/1
  Relational9/1 -> Logical/1
  Logical/1 -> En/1
  [reg_numTrainingSyms] -> Relational1/2, AddSub1/1
  Relational1/1 -> Logical1/1, Logical3/1
  AddSub1/1 -> Relational2/2, Relational3/2
  [reg_numBaseRateSyms] -> AddSub1/2
  Relational2/1 -> Logical1/2
  LSB/1 -> Inverter/1
  Logical1/1 -> Logical/2
  Inverter/1 -> Logical1/3
  Relational3/1 -> Logical2/1
  [TxReg_Alamouti_Mode] -> Logical3/2
  Logical3/1 -> Logical2/2
  Logical2/1 -> Logical/3

== system ofdm_txrx_supermimo_coded/OFDM Tx MIMO/TxControl/Posedge1 ==
  D : Inport  OutDataType=sfix(16)  OutScaling=2^0
  Delay : xbsIndex_r4/Delay  en=off  latency=1  dbl_ovrd=off  reg_retiming=off  block_type=delay
      settings: infoedit=Hardware notes: A delay line is a chain, each link of which is an SRL16 followed by a flip-flop. If register retiming is enabled, the delay line is a chain of flip-flops.  xl_area=[1 1 0 0 0 0 0]  block_version=VER_STRING_GOES_HERE
  Inverter : xbsIndex_r4/Inverter  en=off  latency=0  dbl_ovrd=off  block_type=inv
      settings: xl_area=[0 0 0 0 0 0 0]  block_version=VER_STRING_GOES_HERE
  Logical : xbsIndex_r4/Logical  logical_function=AND  inputs=2  en=off  latency=0  precision=Full  arith_type=Unsigned  n_bits=8  bin_pt=2  align_bp=on  dbl_ovrd=off  block_type=logical
      settings: xl_area=[1 0 0 1 0 0 0]  block_version=VER_STRING_GOES_HERE
  Q : Outport  OutDataType=sfix(16)  OutScaling=2^0
  wires:
  Logical/1 -> Q/1
  D/1 -> Delay/1, Logical/1
  Inverter/1 -> Logical/2
  Delay/1 -> Inverter/1

== system ofdm_txrx_supermimo_coded/OFDM Tx MIMO/TxControl/Posedge2 ==
  D : Inport  OutDataType=sfix(16)  OutScaling=2^0
  Delay : xbsIndex_r4/Delay  en=off  latency=1  dbl_ovrd=off  reg_retiming=off  block_type=delay
      settings: infoedit=Hardware notes: A delay line is a chain, each link of which is an SRL16 followed by a flip-flop. If register retiming is enabled, the delay line is a chain of flip-flops.  xl_area=[1 1 0 0 0 0 0]  block_version=VER_STRING_GOES_HERE
  Inverter : xbsIndex_r4/Inverter  en=off  latency=0  dbl_ovrd=off  block_type=inv
      settings: xl_area=[0 0 0 0 0 0 0]  block_version=VER_STRING_GOES_HERE
  Logical : xbsIndex_r4/Logical  logical_function=AND  inputs=2  en=off  latency=0  precision=Full  arith_type=Unsigned  n_bits=1  bin_pt=0  align_bp=on  dbl_ovrd=off  block_type=logical
      settings: xl_area=[1 0 0 1 0 0 0]  block_version=VER_STRING_GOES_HERE
  Q : Outport  OutDataType=sfix(16)  OutScaling=2^0
  wires:
  Delay/1 -> Inverter/1
  Inverter/1 -> Logical/2
  D/1 -> Logical/1, Delay/1
  Logical/1 -> Q/1

== system ofdm_txrx_supermimo_coded/OFDM Tx MIMO/TxControl/Start/Done Control ==
  TxDone : Inport  OutDataType=sfix(16)  OutScaling=2^0
  Start : Inport  Port=2  OutDataType=sfix(16)  OutScaling=2^0
  Constant2 : xbsIndex_r4/Constant  arith_type=Boolean  const=1  n_bits=1  bin_pt=0  explicit_period=off  period=1  equ=P=C  opselect=C  inp2=PCIN>>17  opr=+  inp1=P  carry=CIN  dbl_ovrd=off  block_type=constant
      settings: block_version=VER_STRING_GOES_HERE
  Convert1 : xbsIndex_r4/Convert  arith_type=Boolean  n_bits=16  bin_pt=14  quantization=Truncate  overflow=Wrap  latency=0  dbl_ovrd=off  pipeline=off  block_type=convert
      settings: block_version=8.2.02
  Convert2 : xbsIndex_r4/Convert  arith_type=Boolean  n_bits=16  bin_pt=14  quantization=Truncate  overflow=Wrap  latency=0  dbl_ovrd=off  pipeline=off  block_type=convert
      settings: block_version=8.2.02
  Inverter : xbsIndex_r4/Inverter  en=off  latency=0  dbl_ovrd=off  block_type=inv
      settings: block_version=8.2.02
  Logical1 : xbsIndex_r4/Logical  logical_function=OR  inputs=2  en=off  latency=0  precision=Full  arith_type=Unsigned  n_bits=8  bin_pt=2  align_bp=on  dbl_ovrd=off  block_type=logical
      settings: xl_area=[1 0 0 1 0 0 0]  block_version=VER_STRING_GOES_HERE
  Logical2 : xbsIndex_r4/Logical  logical_function=OR  inputs=2  en=off  latency=0  precision=Full  arith_type=Unsigned  n_bits=8  bin_pt=2  align_bp=on  dbl_ovrd=off  block_type=logical
      settings: xl_area=[1 0 0 1 0 0 0]  block_version=VER_STRING_GOES_HERE
  Logical3 : xbsIndex_r4/Logical  logical_function=OR  inputs=2  en=off  latency=0  precision=Full  arith_type=Unsigned  n_bits=8  bin_pt=2  align_bp=on  dbl_ovrd=off  block_type=logical
      settings: xl_area=[1 0 0 1 0 0 0]  block_version=VER_STRING_GOES_HERE
  Logical4 : xbsIndex_r4/Logical  logical_function=AND  inputs=2  en=off  latency=0  precision=Full  arith_type=Unsigned  n_bits=8  bin_pt=2  align_bp=on  dbl_ovrd=off  block_type=logical
      settings: xl_area=[1 0 0 1 0 0 0]  block_version=VER_STRING_GOES_HERE
  Posedge1 : Subsystem
  Posedge2 : Subsystem
  Posedge3 : Subsystem
  Posedge4 : Subsystem
  S-R Latch : Subsystem
  S-R Latch1 : Subsystem
  Tx_PktDone : xbsIndex_r4/Gateway Out  hdl_port=on  timing_constraint=None  locs_specified=off  LOCs={}  block_type=gatewayout
      settings: infoedit="Gateway out block.  Converts Xilinx fixed point inputs into ouputs of type Simulink integer, double, or fixed point.<P><P>Hardware notes:  In hardware these blocks become top level output ports or are discarded, depending on how they are configured."  xl_area=[0 0 0 0 1 0 0]  block_version=VER_STRING_GOES_HERE
  Up Sample1 : xbsIndex_r4/Up Sample  sample_ratio=2  copy_samples=on  dbl_ovrd=off  block_type=usamp
      settings: infoedit="Up samples input data.  Inserted values  can be zeros or copies of the most recent input sample.<P><P>Hardware notes: No hardware is needed if inserted values are copies of the input sample; otherwise, a mux and single bit flip-flop are used."  block_version=VER_STRING_GOES_HERE
  Up Sample2 : xbsIndex_r4/Up Sample  sample_ratio=2  copy_samples=on  dbl_ovrd=off  block_type=usamp
      settings: infoedit="Up samples input data.  Inserted values  can be zeros or copies of the most recent input sample.<P><P>Hardware notes: No hardware is needed if inserted values are copies of the input sample; otherwise, a mux and single bit flip-flop are used."  block_version=VER_STRING_GOES_HERE
  Up Sample3 : xbsIndex_r4/Up Sample  sample_ratio=2  copy_samples=on  dbl_ovrd=off  block_type=usamp
      settings: infoedit="Up samples input data.  Inserted values  can be zeros or copies of the most recent input sample.<P><P>Hardware notes: No hardware is needed if inserted values are copies of the input sample; otherwise, a mux and single bit flip-flop are used."  block_version=VER_STRING_GOES_HERE
  Up Sample4 : xbsIndex_r4/Up Sample  sample_ratio=4  copy_samples=on  dbl_ovrd=off  block_type=usamp
      settings: infoedit="Up samples input data.  Inserted values  can be zeros or copies of the most recent input sample.<P><P>Hardware notes: No hardware is needed if inserted values are copies of the input sample; otherwise, a mux and single bit flip-flop are used."  block_version=VER_STRING_GOES_HERE
  TxStart : Outport  OutDataType=sfix(16)  OutScaling=2^0
  tag scope: regTx_PktRunning=global, regTx_PktRunningEn=global
  wires:
  Start/1 -> Posedge2/1(D)
  TxDone/1 -> Posedge3/1(D)
  Logical1/1 -> S-R Latch/2(R), Up Sample3/1
  [TxReset] -> Logical1/2
  S-R Latch/1(Q) -> Inverter/1
  Constant2/1 -> [regTx_PktRunningEn]
  Inverter/1 -> Convert2/1
  Logical4/1 -> TxStart/1
  Posedge3/1(Q) -> Logical1/1, Up Sample2/1
  Convert1/1 -> Logical4/1
  Convert2/1 -> Logical4/2
  Posedge2/1(Q) -> S-R Latch/1(S), Convert1/1, Up Sample1/1
  Up Sample2/1 -> Tx_PktDone/1
  Logical2/1 -> S-R Latch1/1(S)
  [TxEn_Output] -> Posedge4/1(D)
  Posedge4/1(Q) -> Logical2/1
  S-R Latch1/1(Q) -> [regTx_PktRunning]
  Up Sample1/1 -> Logical2/2
  Up Sample3/1 -> Logical3/1
  Logical3/1 -> S-R Latch1/2(R)
  [AF_TxDone] -> Posedge1/1(D)
  Posedge1/1(Q) -> Up Sample4/1
  Up Sample4/1 -> Logical3/2

== system ofdm_txrx_supermimo_coded/OFDM Tx MIMO/TxStart/Copy_Downsample ==
  D : Inport  OutDataType=sfix(16)  OutScaling=2^0
  Delay : xbsIndex_r4/Delay  en=off  latency=1  dbl_ovrd=off  reg_retiming=off  block_type=delay
      settings: infoedit=Hardware notes: A delay line is a chain, each link of which is an SRL16 followed by a flip-flop. If register retiming is enabled, the delay line is a chain of flip-flops.  block_version=VER_STRING_GOES_HERE
  Down Sample : xbsIndex_r4/Down Sample  sample_ratio=2  sample_phase="Last Value of Frame  (most efficient)"  en=off  latency=1  dbl_ovrd=off  block_type=dsamp
      settings: block_version=VER_STRING_GOES_HERE
  Logical : xbsIndex_r4/Logical  logical_function=OR  inputs=2  en=off  latency=0  precision=Full  arith_type=Unsigned  n_bits=16  bin_pt=0  align_bp=on  dbl_ovrd=off  block_type=logical
      settings: block_version=VER_STRING_GOES_HERE
  Q : Outport  OutDataType=sfix(16)  OutScaling=2^0
  wires:
  Down Sample/1 -> Q/1
  Logical/1 -> Down Sample/1
  D/1 -> Logical/2, Delay/1
  Delay/1 -> Logical/1

== system ofdm_txrx_supermimo_coded/OFDM Tx MIMO/TxStart/posedge1 ==
  D : Inport  OutDataType=sfix(16)  OutScaling=2^0
  Delay : xbsIndex_r4/Delay  en=off  latency=1  dbl_ovrd=off  reg_retiming=off  block_type=delay
      settings: infoedit=Hardware notes: A delay line is a chain, each link of which is an SRL16 followed by a flip-flop. If register retiming is enabled, the delay line is a chain of flip-flops.  xl_area=[1 1 0 0 0 0 0]  block_version=VER_STRING_GOES_HERE
  Inverter : xbsIndex_r4/Inverter  en=off  latency=0  dbl_ovrd=off  block_type=inv
      settings: xl_area=[0 0 0 0 0 0 0]  block_version=VER_STRING_GOES_HERE
  Logical1 : xbsIndex_r4/Logical  logical_function=AND  inputs=2  en=off  latency=0  precision=Full  arith_type=Unsigned  n_bits=8  bin_pt=2  align_bp=on  dbl_ovrd=off  block_type=logical
      settings: xl_area=[1 0 0 1 0 0 0]  block_version=VER_STRING_GOES_HERE
  Q : Outport  OutDataType=sfix(16)  OutScaling=2^0
  wires:
  Inverter/1 -> Logical1/1
  D/1 -> Logical1/2, Delay/1
  Delay/1 -> Inverter/1
  Logical1/1 -> Q/1

== system ofdm_txrx_supermimo_coded/Pkt Buffer BRAM Interface/Pkt Buffer Addressing/Rx Word Addr Generation ==
  note: Supports two modes for dividing the big PLB BRAM packet buffer:
      |
      | BigPktMode = 1 uses 16KB sub-buffers (4 buffers in 64KB)
      | BigPktMode = 0 uses 2KB sub-buffers (32 buffers in 64KB)
  2LSB+8 : xbsIndex_r4/Slice  nbits=2  boolean_output=off  mode=Lower Bit Location + Width  bit1=0  base1=MSB of Input  bit0=8  base0=LSB of Input  dbl_ovrd=off  block_type=slice
      settings: infoedit="Extracts a given range of bits from each input sample and presents it at the output.  The output type is ordinarily unsigned with binary point at zero, but can be Boolean when the slice is one bit wide.<P><P>Hardware notes: In hardware this block costs nothing."  block_version=VER_STRING_GOES_HERE
  5LSB+8 : xbsIndex_r4/Slice  nbits=5  boolean_output=off  mode=Lower Bit Location + Width  bit1=0  base1=MSB of Input  bit0=8  base0=LSB of Input  dbl_ovrd=off  block_type=slice
      settings: infoedit="Extracts a given range of bits from each input sample and presents it at the output.  The output type is ordinarily unsigned with binary point at zero, but can be Boolean when the slice is one bit wide.<P><P>Hardware notes: In hardware this block costs nothing."  block_version=VER_STRING_GOES_HERE
  "8LSB " : xbsIndex_r4/Slice  nbits=8  boolean_output=off  mode=Lower Bit Location + Width  bit1=0  base1=MSB of Input  bit0=0  base0=LSB of Input  dbl_ovrd=off  block_type=slice
      settings: infoedit="Extracts a given range of bits from each input sample and presents it at the output.  The output type is ordinarily unsigned with binary point at zero, but can be Boolean when the slice is one bit wide.<P><P>Hardware notes: In hardware this block costs nothing."  block_version=VER_STRING_GOES_HERE
  Concat7 : xbsIndex_r4/Concat  num_inputs=2  dbl_ovrd=off  block_type=concat
      settings: block_version=8.2.02
  Concat8 : xbsIndex_r4/Concat  num_inputs=2  dbl_ovrd=off  block_type=concat
      settings: block_version=8.2.02
  Mux1 : xbsIndex_r4/Mux  inputs=2  en=off  latency=0  precision=Full  arith_type=Unsigned  n_bits=16  bin_pt=14  quantization=Truncate  overflow=Wrap  dbl_ovrd=off  block_type=mux
      settings: block_version=8.2.01
  Word Addr : Outport
  wires:
  Concat8/1 -> Mux1/2
  Concat7/1 -> Mux1/3
  5LSB+8/1 -> Concat8/1
  "8LSB "/1 -> Concat8/2
  [regTxRx_PktBuffOffsets] -> 5LSB+8/1, 2LSB+8/1
  2LSB+8/1 -> Concat7/1
  [BRAM_Rx_WrAddr] -> "8LSB "/1, Concat7/2
  [regTxRx_BigPktBufMode] -> Mux1/1
  Mux1/1 -> Word Addr/1

== system ofdm_txrx_supermimo_coded/Pkt Buffer BRAM Interface/Pkt Buffer Addressing/Tx Word Addr Generation ==
  note: The Tx Pkt Buf number can come from three places:
      | -User code, via the PktBuffOffsets register (the "old" flow)
      | -The autoReply actionID, specifying a template pktBuffer
      | -The autoReply header translator, specifying a potentially different
      |    buffer for just the header bytes.
      |
      | In a single pkt Tx event, the buffer selection is either:
      | -Software-only, written to the register and cosntant throughout the Tx
      |    or
      | -Hardware-only, using the actionID buffer number except where overridden
      |   by the header translator
  note: Truth table:
      | x = AutoReply triggered this transmission
      | y = Header translator should be used for this byte
      | z = 2-bit mux select
      |
      | x y z
      | 0 0 00 (no autoTx; use the register)
      | 0 1 x (invalid inputs - should never occur)
      | 1 0 01 (autoTx, but not a translated header byte)
      | 1 1 10 (autoTx and translated header byte)
  5LSB : xbsIndex_r4/Slice  nbits=5  boolean_output=off  mode=Lower Bit Location + Width  bit1=0  base1=MSB of Input  bit0=0  base0=LSB of Input  dbl_ovrd=off  block_type=slice
      settings: infoedit="Extracts a given range of bits from each input sample and presents it at the output.  The output type is ordinarily unsigned with binary point at zero, but can be Boolean when the slice is one bit wide.<P><P>Hardware notes: In hardware this block costs nothing."  block_version=VER_STRING_GOES_HERE
  Concat1 : xbsIndex_r4/Concat  num_inputs=2  dbl_ovrd=off  block_type=concat
      settings: block_version=8.2.02
  Logical2 : xbsIndex_r4/Logical  logical_function=AND  inputs=2  en=off  latency=0  precision=Full  arith_type=Unsigned  n_bits=16  bin_pt=0  align_bp=on  dbl_ovrd=off  block_type=logical
  Logical3 : xbsIndex_r4/Logical  logical_function=XOR  inputs=2  en=off  latency=0  precision=Full  arith_type=Unsigned  n_bits=16  bin_pt=0  align_bp=on  dbl_ovrd=off  block_type=logical
  Mux1 : xbsIndex_r4/Mux  inputs=3  en=off  latency=0  precision=Full  arith_type=Unsigned  n_bits=16  bin_pt=14  quantization=Truncate  overflow=Wrap  dbl_ovrd=off  block_type=mux
      settings: block_version=8.2.01
  Mux2 : xbsIndex_r4/Mux  inputs=2  en=off  latency=0  precision=Full  arith_type=Unsigned  n_bits=16  bin_pt=14  quantization=Truncate  overflow=Wrap  dbl_ovrd=off  block_type=mux
      settings: block_version=8.2.01
  WordAddrGen : Subsystem
  Word Addr : Outport
  tag scope: PreSpin_TxBufIndex=global
  wires:
  [RxAutoReply_TransHdr_BufOveride] -> Logical3/1, Logical2/2
  [RxAutoReply_AutoTx] -> Logical3/2, Logical2/1, Mux2/1
  Logical3/1 -> Concat1/2
  Logical2/1 -> Concat1/1
  [regTxRx_PktBuffOffsets] -> 5LSB/1
  5LSB/1 -> Mux1/2, Mux2/2
  [RxAutoReply_Action_BufNum] -> Mux1/3, Mux2/3
  [RxAutoReply_TransHdr_BufNum] -> Mux1/4
  Concat1/1 -> Mux1/1
  WordAddrGen/1(Word Addr) -> Word Addr/1
  Mux2/1 -> [PreSpin_TxBufIndex]
  Mux1/1 -> WordAddrGen/1(Buffer Ind)

== system ofdm_txrx_supermimo_coded/Memory-mapped Registers/Rx Registers/Pkt Detector Registers/Read-Write Register1 ==
  Constant : xbsIndex_r4/Constant  arith_type=Boolean  const=1  n_bits=16  bin_pt=14  explicit_period=on  period=sampPeriod  equ=P=C  opselect=C  inp2=PCIN>>17  opr=+  inp1=P  carry=CIN  dbl_ovrd=off  block_type=constant
      settings: block_version=8.2.02
  From Register : xbsIndex_r4/From Register  shared_memory_name='pktDet_controlBits_w'  init=120  period=sampPeriod  ownership=Locally owned and initialized  arith_type=Unsigned  n_bits=32  bin_pt=0  dbl_ovrd=off  block_type=fromreg
      settings: AttributesFormatString=From Register\n<< %<shared_memory_name> >>\n%<n_bits>\n%<period>\n%<bin_pt>\n%<arith_type>\n%<ownership>\n%<init>  infoedit="Register block that reads data to a shared memory register.  Delay of one sample period."  block_version=8.2.02
  Terminator : Terminator
  To Register : xbsIndex_r4/To Register  shared_memory_name='pktDet_controlBits_r'  init=120  ownership=Locally owned and initialized  explicit_data_type=on  arith_type=Unsigned  n_bits=32  bin_pt=0  dbl_ovrd=off  block_type=toreg
      settings: AttributesFormatString=To Register\n<< %<shared_memory_name> >>  infoedit="Register block that writes data to a shared memory register.  Delay of one sample period."  block_version=8.2.02
  D : Outport  OutDataType=sfix(16)  OutScaling=2^0
  wires:
  From Register/1 -> D/1, To Register/1
  Constant/1 -> To Register/2
  To Register/1 -> Terminator/1
